simulink model "vegas01_4tap_1024ch_220_r8b"  (Simulink 7.5)
settings: Solver=ode45  StartTime=0.0  StopTime=128000  RelTol=1e-3
libraries: xbsIndex_r4 x12580, monroe_library x80, xbsReplacements_r4 x42, xps_library x34, casper_library_misc x27, dspsigops x16, simulink x7
(part 9 of 91)

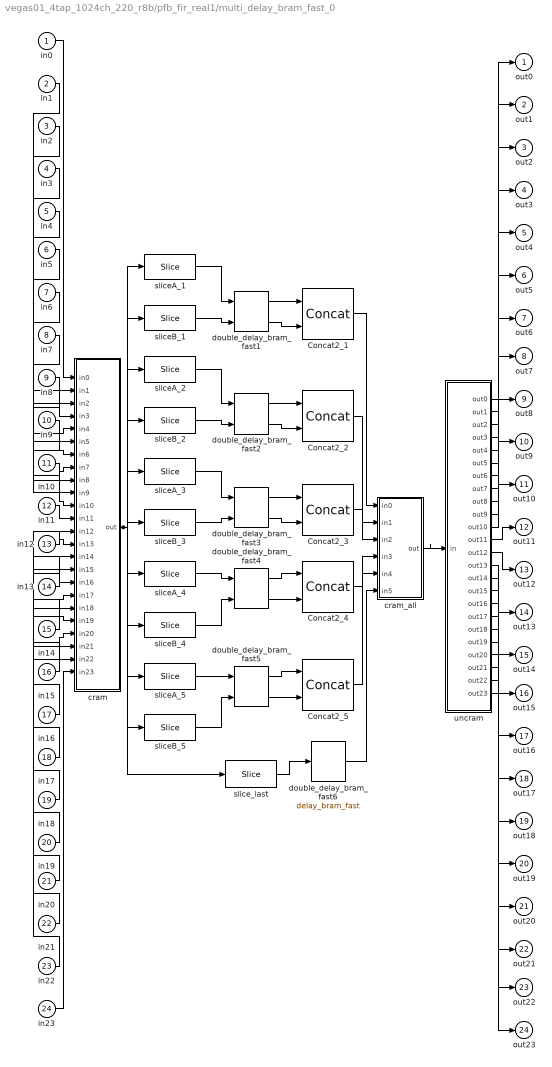
== system vegas01_4tap_1024ch_220_r8b/pfb_fir_real1/multi_delay_bram_fast_0 ==
  in0 : Inport
  in1 : Inport  Port=2
  in2 : Inport  Port=3
  in3 : Inport  Port=4
  in4 : Inport  Port=5
  in5 : Inport  Port=6
  in6 : Inport  Port=7
  in7 : Inport  Port=8
  in8 : Inport  Port=9
  in9 : Inport  Port=10
  in10 : Inport  Port=11
  in11 : Inport  Port=12
  in12 : Inport  Port=13
  in13 : Inport  Port=14
  in14 : Inport  Port=15
  in15 : Inport  Port=16
  in16 : Inport  Port=17
  in17 : Inport  Port=18
  in18 : Inport  Port=19
  in19 : Inport  Port=20
  in20 : Inport  Port=21
  in21 : Inport  Port=22
  in22 : Inport  Port=23
  in23 : Inport  Port=24
  Concat2_{1..5} (5 alike) : xbsIndex_r4/Concat  num_inputs=2  dbl_ovrd=off  block_type=concat
  cram : Subsystem
  cram_all : Subsystem
  double_delay_bram_fast{1..5} (5 alike) : monroe_library/double_delay_bram_fast  SystemSampleTime=-1  delay_len=256  bram_latency=2  register_counter=1
  double_delay_bram_fast6 : monroe_library/delay_bram_fast  SystemSampleTime=-1  delay_len=256  bram_latency=2  memory_type=Block RAM  register_counter=1
  sliceA_1 : xbsIndex_r4/Slice  nbits=18  boolean_output=off  mode=Upper Bit Location + Width  bit1=-0  base1=MSB of Input  bit0=-0  base0=MSB of Input  dbl_ovrd=off  block_type=slice
  sliceA_2 : xbsIndex_r4/Slice  nbits=18  boolean_output=off  mode=Upper Bit Location + Width  bit1=-36  base1=MSB of Input  bit0=-36  base0=MSB of Input  dbl_ovrd=off  block_type=slice
  sliceA_3 : xbsIndex_r4/Slice  nbits=18  boolean_output=off  mode=Upper Bit Location + Width  bit1=-72  base1=MSB of Input  bit0=-72  base0=MSB of Input  dbl_ovrd=off  block_type=slice
  sliceA_4 : xbsIndex_r4/Slice  nbits=18  boolean_output=off  mode=Upper Bit Location + Width  bit1=-108  base1=MSB of Input  bit0=-108  base0=MSB of Input  dbl_ovrd=off  block_type=slice
  sliceA_5 : xbsIndex_r4/Slice  nbits=18  boolean_output=off  mode=Upper Bit Location + Width  bit1=-144  base1=MSB of Input  bit0=-144  base0=MSB of Input  dbl_ovrd=off  block_type=slice
  sliceB_1 : xbsIndex_r4/Slice  nbits=18  boolean_output=off  mode=Upper Bit Location + Width  bit1=-18  base1=MSB of Input  bit0=-18  base0=MSB of Input  dbl_ovrd=off  block_type=slice
  sliceB_2 : xbsIndex_r4/Slice  nbits=18  boolean_output=off  mode=Upper Bit Location + Width  bit1=-54  base1=MSB of Input  bit0=-54  base0=MSB of Input  dbl_ovrd=off  block_type=slice
  sliceB_3 : xbsIndex_r4/Slice  nbits=18  boolean_output=off  mode=Upper Bit Location + Width  bit1=-90  base1=MSB of Input  bit0=-90  base0=MSB of Input  dbl_ovrd=off  block_type=slice
  sliceB_4 : xbsIndex_r4/Slice  nbits=18  boolean_output=off  mode=Upper Bit Location + Width  bit1=-126  base1=MSB of Input  bit0=-126  base0=MSB of Input  dbl_ovrd=off  block_type=slice
  sliceB_5 : xbsIndex_r4/Slice  nbits=18  boolean_output=off  mode=Upper Bit Location + Width  bit1=-162  base1=MSB of Input  bit0=-162  base0=MSB of Input  dbl_ovrd=off  block_type=slice
  slice_last : xbsIndex_r4/Slice  nbits=12  boolean_output=off  mode=Lower Bit Location + Width  bit1=0  base1=LSB of Input  bit0=0  base0=LSB of Input  dbl_ovrd=off  block_type=slice
  uncram : Subsystem
  out0 : Outport
  out1 : Outport  Port=2
  out2 : Outport  Port=3
  out3 : Outport  Port=4
  out4 : Outport  Port=5
  out5 : Outport  Port=6
  out6 : Outport  Port=7
  out7 : Outport  Port=8
  out8 : Outport  Port=9
  out9 : Outport  Port=10
  out10 : Outport  Port=11
  out11 : Outport  Port=12
  out12 : Outport  Port=13
  out13 : Outport  Port=14
  out14 : Outport  Port=15
  out15 : Outport  Port=16
  out16 : Outport  Port=17
  out17 : Outport  Port=18
  out18 : Outport  Port=19
  out19 : Outport  Port=20
  out20 : Outport  Port=21
  out21 : Outport  Port=22
  out22 : Outport  Port=23
  out23 : Outport  Port=24
  wires:
  cram/1(out) -> slice_last/1, sliceB_5/1, sliceA_5/1, sliceB_4/1, sliceA_4/1, sliceB_3/1, sliceA_3/1, sliceB_2/1, sliceA_2/1, sliceB_1/1, sliceA_1/1
  uncram/24(out23) -> out23/1
  uncram/23(out22) -> out22/1
  uncram/22(out21) -> out21/1
  uncram/21(out20) -> out20/1
  uncram/20(out19) -> out19/1
  uncram/19(out18) -> out18/1
  uncram/18(out17) -> out17/1
  uncram/17(out16) -> out16/1
  uncram/16(out15) -> out15/1
  uncram/15(out14) -> out14/1
  uncram/14(out13) -> out13/1
  uncram/13(out12) -> out12/1
  uncram/12(out11) -> out11/1
  uncram/11(out10) -> out10/1
  uncram/10(out9) -> out9/1
  uncram/9(out8) -> out8/1
  uncram/8(out7) -> out7/1
  uncram/7(out6) -> out6/1
  uncram/6(out5) -> out5/1
  uncram/5(out4) -> out4/1
  uncram/4(out3) -> out3/1
  uncram/3(out2) -> out2/1
  uncram/2(out1) -> out1/1
  uncram/1(out0) -> out0/1
  cram_all/1(out) -> uncram/1(in)
  double_delay_bram_fast6/1 -> cram_all/6(in5)
  Concat2_5/1 -> cram_all/5(in4)
  Concat2_4/1 -> cram_all/4(in3)
  Concat2_3/1 -> cram_all/3(in2)
  Concat2_2/1 -> cram_all/2(in1)
  Concat2_1/1 -> cram_all/1(in0)
  slice_last/1 -> double_delay_bram_fast6/1
  double_delay_bram_fast5/2 -> Concat2_5/2
  double_delay_bram_fast5/1 -> Concat2_5/1
  sliceB_5/1 -> double_delay_bram_fast5/2
  sliceA_5/1 -> double_delay_bram_fast5/1
  double_delay_bram_fast4/2 -> Concat2_4/2
  double_delay_bram_fast4/1 -> Concat2_4/1
  sliceB_4/1 -> double_delay_bram_fast4/2
  sliceA_4/1 -> double_delay_bram_fast4/1
  double_delay_bram_fast3/2 -> Concat2_3/2
  double_delay_bram_fast3/1 -> Concat2_3/1
  sliceB_3/1 -> double_delay_bram_fast3/2
  sliceA_3/1 -> double_delay_bram_fast3/1
  double_delay_bram_fast2/2 -> Concat2_2/2
  double_delay_bram_fast2/1 -> Concat2_2/1
  sliceB_2/1 -> double_delay_bram_fast2/2
  sliceA_2/1 -> double_delay_bram_fast2/1
  double_delay_bram_fast1/2 -> Concat2_1/2
  double_delay_bram_fast1/1 -> Concat2_1/1
  sliceB_1/1 -> double_delay_bram_fast1/2
  sliceA_1/1 -> double_delay_bram_fast1/1
  in23/1 -> cram/24(in23)
  in22/1 -> cram/23(in22)
  in21/1 -> cram/22(in21)
  in20/1 -> cram/21(in20)
  in19/1 -> cram/20(in19)
  in18/1 -> cram/19(in18)
  in17/1 -> cram/18(in17)
  in16/1 -> cram/17(in16)
  in15/1 -> cram/16(in15)
  in14/1 -> cram/15(in14)
  in13/1 -> cram/14(in13)
  in12/1 -> cram/13(in12)
  in11/1 -> cram/12(in11)
  in10/1 -> cram/11(in10)
  in9/1 -> cram/10(in9)
  in8/1 -> cram/9(in8)
  in7/1 -> cram/8(in7)
  in6/1 -> cram/7(in6)
  in5/1 -> cram/6(in5)
  in4/1 -> cram/5(in4)
  in3/1 -> cram/4(in3)
  in2/1 -> cram/3(in2)
  in1/1 -> cram/2(in1)
  in0/1 -> cram/1(in0)

== system vegas01_4tap_1024ch_220_r8b/pfb_fir_real3/coeff_gen_0_7 ==
  sync : Inport
  Counter0 : xbsIndex_r4/Counter  cnt_type=Free Running  cnt_to=Inf  operation=Up  start_count=8  cnt_by_val=1  arith_type=Unsigned  n_bits=8  bin_pt=0  load_pin=off  rst=on  en=off  explicit_period=on  period=1  dbl_ovrd=off  use_behavioral_HDL=off  implementation=Fabric  block_type=counter
  Counter1 : xbsIndex_r4/Counter  cnt_type=Free Running  cnt_to=Inf  operation=Down  start_count=247  cnt_by_val=1  arith_type=Unsigned  n_bits=8  bin_pt=0  load_pin=off  rst=on  en=off  explicit_period=on  period=1  dbl_ovrd=off  use_behavioral_HDL=off  implementation=Fabric  block_type=counter
  Delay1 : xbsIndex_r4/Delay  rst=off  infoeditControl=Selection of Reset will increase slice count due to use of real FFs and instead of SRLs  en=off  latency=2  dbl_ovrd=off  reg_retiming=off  block_type=delay
  Delay2 : xbsIndex_r4/Delay  rst=off  infoeditControl=Selection of Reset will increase slice count due to use of real FFs and instead of SRLs  en=off  latency=1  dbl_ovrd=off  reg_retiming=off  block_type=delay
  Delay3 : xbsIndex_r4/Delay  rst=off  infoeditControl=Selection of Reset will increase slice count due to use of real FFs and instead of SRLs  en=off  latency=1  dbl_ovrd=off  reg_retiming=off  block_type=delay
  Delay4 : xbsIndex_r4/Delay  rst=off  infoeditControl=Selection of Reset will increase slice count due to use of real FFs and instead of SRLs  en=off  latency=2  dbl_ovrd=off  reg_retiming=off  block_type=delay
  coeff_ram_1 : xbsIndex_r4/Dual Port RAM  depth=256  initVector=[-3.1185374660155e-18 -0.000156568793162539 -0.000313829844983494 -0.000471865598633262 -0.000630759038071172 -0.000790593639144139 -0.000951453320173921 -0.00111342239204917 -0.00127658550783793 -0.00144102761193718 -0.00160683388877549 -0.00177408971108584 -0.00194288058776502 -0.00211329211133704 -0.00228540990503756 -0.00245931956953669 -0.00263510662931799 -0.00281285647873119 -0.00299265432773675 -0.00317458514736013 -0.00335873361487434 -0.00354518405872877 -0.00373402040324324 -0.00392532611308567 -0.00411918413755233 -0.00431567685466965 -0.00451488601513651 -0.00471689268612641 -0.00492177719496865 -0.00512961907272809 -0.00534049699770283 -0.00555448873885967 -0.00577167109922673 -0.00599211985926327 -0.00621590972022655 -0.00644311424755544 -0.00667380581429108 -0.00690805554455435 -0.00714593325710056 -0.0073875074089712 -0.00763284503926322 -0.00788201171303579 -0.00813507146537516 -0.00839208674563745 -0.00865311836189 -0.00891822542557158 -0.0091874652963913 -0.00946089352748732 -0.00973856381086471 -0.0100205279231336 -0.0103068356715673 -0.0105975348405007 -0.010892671138089 -0.0111922881434479 -0.0114964272541938 -0.0118051276344046 -0.012118426163022 -0.0124363573827139 -0.0127589534492176 -0.0130862440811825 -0.0134182565105339 -0.0137550154333747 -0.0140965429614476 -0.0144428585741738 -0.0147939790712908 -0.0151499185261055 -0.015510688239384 -0.0158762966938947 -0.0162467495096255 -0.0166220493996923 -0.0170021961269577 -0.0173871864613782 -0.0177770141380983 -0.0181716698163081 -0.0185711410388843 -0.0189754121928306 -0.019384464470535 -0.0197982758318616 -0.0202168209670949 -0.0206400712607505 -0.0210679947562723 -0.02150055612163 -0.021937716615834 -0.0223794340563839 -0.022825662787666 -0.0232763536503145 -0.0237314539515533 -0.0241909074365316 -0.0246546542606692 -0.0251226309630254 -0.0255947704407058 -0.0260710019243209 -0.0265512509545099 -0.0270354393595443 -0.0275234852340214 -0.0280153029186648 -0.02851080298124 -0.0290098921986007 -0.0295124735398754 -0.030018446150807 -0.0305277053392568 -0.0310401425618828 -0.0315556454120042 -0.032074097608661 -0.0325953789868802 -0.0331193654891569 -0.0336459291581604 -0.0341749381306741 -0.0347062566327772 -0.0352397449762773 -0.0357752595564017 -0.0363126528507542 -0.0368517734195453 -0.0373924659071026 -0.0379345710446673 -0.0384779256544837 -0.0390223626551864 -0.0395677110684921 -0.0401137960271989 -0.0406604387844996 -0.0412074567246124 -0.0417546633747318 -0.042301868418306 -0.0428488777096403 -0.0433954932898318 -0.0439415134040368 -0.0444867325200723 -0.0450309413483536 -0.0455739268631693 -0.0461154723252939 -0.0466553573059384 -0.0471933577120388 -0.0477292458128818 -0.0482627902680681 -0.0487937561568089 -0.0493219050085576 -0.0498469948349719 -0.0503687801632044 -0.0508870120705198 -0.0514014382202336 -0.05191180289897 -0.0524178470552337 -0.0529193083392921 -0.0534159211443621 -0.0539074166490959 -0.0543935228613613 -0.0548739646633084 -0.0553484638577182 -0.0558167392156239 -0.0562785065251991 -0.0567334786419044 -0.0571813655398841 -0.0576218743646051 -0.0580547094867273 -0.0584795725571982 -0.0588961625635602 -0.0593041758874615 -0.0597033063633591 -0.0600932453384034 -0.0604736817334929 -0.0608443021054873 -0.0612047907105668 -0.0615548295687251 -0.0618940985293826 -0.0622222753381076 -0.0625390357044313 -0.0628440533707418 -0.0631370001822442 -0.0634175461579711 -0.0636853595628278 -0.0639401069806586 -0.0641814533883164 -0.0644090622307204 -0.0646225954968852 -0.0648217137969047 -0.0650060764398726 -0.0651753415127238 -0.0653291659599763 -0.0654672056643582 -0.0655891155282986 -0.0656945495562658 -0.0657831609379319 -0.0658546021321458 -0.0659085249516942 -0.0659445806488293 -0.0659624200015456 -0.0659616934005828 -0.0659420509371349 -0.0659031424912438 -0.0658446178208569 -0.0657661266515254 -0.0656673187667229 -0.0655478440987611 -0.0654073528202804 -0.0652454954362926 -0.0650619228767521 -0.0648562865896345 -0.064628238634496 ...(+1157 chars)]  distributed_mem=Block RAM  init_a=0  init_b=0  rst_a=off  rst_b=off  en_a=off  en_b=off  latency=2  write_mode_A=No Read On Write  write_mode_B=No Read On Write  dbl_ovrd=off  optimize=Area  use_rpm=on  block_type=dpram
  coeff_ram_2 : xbsIndex_r4/Dual Port RAM  depth=256  initVector=[2.10535666547559e-17 0.00212900955948074 0.00429668515967571 0.00650315122411092 0.00874852581948401 0.0110329205850847 0.0133564406634076 0.0157191846319806 0.0181212444364309 0.0205627053248104 0.023043645783203 0.0255641374726353 0.0281242451673111 0.0307240266941921 0.0333635328739444 0.0360428074632712 0.0387618870986534 0.0415208012415143 0.044319572124833 0.0471582147012203 0.0500367365924796 0.0529551380406698 0.0559134118606878 0.0589115433943901 0.0619495104662685 0.0650272833406989 0.0681448246807793 0.0713020895087723 0.0744990251681692 0.0777355712873909 0.0810116597451406 0.0843272146374221 0.0876821522462404 0.0910763810099955 0.0945098014955858 0.0979823063722314 0.101493780387033 0.105044100342275 0.108633135074489 0.112260745435285 0.115926784273964 0.119631096421919 0.123373518678842 0.127153879800738 0.130972000489756 0.134827693385857 0.138720763060312 0.142651006011044 0.146618210659827 0.150622157351339 0.154662618354086 0.158739357863189 0.162852132005056 0.167000688843928 0.171184768390318 0.175404102611337 0.179658415442912 0.183947422803904 0.188270832612124 0.192628344802252 0.197019651345652 0.201444436272104 0.205902375693431 0.210393137829031 0.214916383033319 0.21947176382507 0.224058924918664 0.22867750325723 0.233327128047692 0.238007420797708 0.242717995354501 0.24745845794558 0.25222840722134 0.257027434299547 0.261855122811695 0.266711048951228 0.271594781523624 0.27650588199834 0.281443904562596 0.286408396177002 0.291398896633021 0.296414938612245 0.301456047747492 0.306521742685707 0.31161153515265 0.316724930019371 0.321861425370457 0.327020512574038 0.33220167635354 0.337404394861171 0.342628139753137 0.347872376266556 0.353136563298071 0.358420153484148 0.363722593283031 0.369043323058348 0.374381777164357 0.379737384032801 0.385109566261371 0.390497740703747 0.395901318561207 0.401319705475786 0.406752301624961 0.412198501817848 0.41765769559289 0.423129267317018 0.428612596286257 0.434107056827774 0.439612018403325 0.445126845714092 0.450650898806897 0.456183533181742 0.461724099900691 0.467271945698036 0.472826413091746 0.478386840496164 0.483952562335939 0.489522909161153 0.495097207763634 0.500674781294419 0.506254949382349 0.511837028253763 0.517420330853274 0.523004166965589 0.52858784333836 0.534170663806028 0.53975192941464 0.545330938547599 0.550906987052345 0.556479368367905 0.562047373653314 0.567610291916857 0.573167410146117 0.578718013438789 0.584261385134243 0.589796806945793 0.595323559093652 0.600840920438539 0.606348168615905 0.611844580170759 0.617329430693046 0.622801994953559 0.628261547040357 0.633707360495634 0.639138708453047 0.644554863775432 0.649955099192899 0.655338687441275 0.660704901400846 0.666053014235387 0.671382299531429 0.676692031437751 0.68198148480504 0.687249935325708 0.692496659673825 0.697720935645132 0.702922042297109 0.708099260089058 0.713251871022173 0.718379158779564 0.723480408866197 0.728554908748728 0.733601947995182 0.738620818414463 0.743610814195645 0.748571232047023 0.753501371334883 0.758400534221968 0.763268025805597 0.768103154255408 0.772905230950694 0.777673570617301 0.782407491464052 0.787106315318659 0.791769367763108 0.796395978268463 0.800985480329079 0.805537211596169 0.810050514010716 0.814524733935677 0.818959222287464 0.823353334666666 0.827706431487968 0.832017878109261 0.836287044959883 0.840513307667989 0.844696047186994 0.848834649921077 0.852928507849702 0.856977018651141 0.860979585824954 0.864935618813407 0.868844533121792 0.87270575043763 0.876518698748711 0.880282812459962 0.8839975325091 0.887662306481051 0.891276588721101 0.894839840446757 0.898351529858287 0.901811132247913 0.905218130107634 0.908572013235644 0.911872278841335 0.915118431648839 0.918309983999099 0.921446455950444 0.92452737537763 0.92755227806934 0.93052070782411 0.933432216544651 0.936286364330567 0.939082719569415 0.941820859026115 0.944500367930665 0.947120840064154 0.949681877843045 0.952183092401712 0.954624103673203 ...(+639 chars)]  distributed_mem=Block RAM  init_a=0  init_b=0  rst_a=off  rst_b=off  en_a=off  en_b=off  latency=2  write_mode_A=No Read On Write  write_mode_B=No Read On Write  dbl_ovrd=off  optimize=Area  use_rpm=on  block_type=dpram
  coeff_ram_3 : xbsIndex_r4/Dual Port RAM  depth=256  initVector=[0.99999996616597 0.999965122732456 0.999862763148446 0.99969290322501 0.999455569174354 0.999150797604323 0.998778635510719 0.998339140267444 0.997832379614464 0.997258431643602 0.996617384782167 0.995909337774408 0.995134399660817 0.994292689755268 0.993384337620019 0.992409483038553 0.991368275986299 0.990260876599208 0.989087455140225 0.987848191963625 0.986543277477267 0.98517291210273 0.983737306233386 0.982236680190373 0.980671264176525 0.979041298228237 0.977347032165284 0.975588725538626 0.973766647576178 0.971881077126593 0.969932302601044 0.967920621913036 0.965846342416255 0.963709780840474 0.961511263225527 0.959251124853375 0.956929710178262 0.954547372755009 0.952104475165433 0.949601388942924 0.947038494495202 0.944416181025259 0.94173484645052 0.938994897320233 0.936196748731109 0.933340824241245 0.930427555782326 0.927457383570154 0.924430756013512 0.921348129621381 0.918209968908548 0.915016746299614 0.911768942031431 0.90846704405399 0.905111547929788 0.901702956731696 0.898241780939348 0.894728538334085 0.891163753892475 0.887547959678431 0.883881694733966 0.88016550496859 0.8763999430474 0.872585568277877 0.868722946495407 0.864812649947588 0.860855257177304 0.85685135290464 0.852801527907634 0.848706378901905 0.844566508419199 0.840382524684857 0.836155041494258 0.831884678088254 0.827572059027625 0.8232178140666 0.818822578025451 0.814386990662214 0.809911696543554 0.805397344914799 0.800844589569198 0.79625408871641 0.791626504850263 0.786962504615826 0.782262758675808 0.777527941576326 0.772758731612076 0.767955810690928 0.763119864197992 0.758251580859177 0.753351652604278 0.74842077442962 0.743459644260307 0.738468962812079 0.73344943345285 0.728401762063917 0.723326656900904 0.718224828454464 0.713096989310765 0.707943854011803 0.70276613891557 0.69756456205611 0.692339843003496 0.687092702723761 0.68182386343882 0.676534048486405 0.671223982180056 0.665894389669201 0.660545996799345 0.655179529972417 0.649795716007294 0.644395282000545 0.638978955187421 0.633547462803117 0.628101531944358 0.622641889431319 0.617169261669924 0.611684374514551 0.606187953131173 0.60068072186097 0.595163404084448 0.589636722086077 0.584101396919502 0.578558148273344 0.57300769433762 0.567450751670829 0.561888035067706 0.556320257427704 0.550748129624207 0.545172360374529 0.5395936561107 0.534012720851096 0.528430256072918 0.522846960585568 0.517263530404928 0.511680658628599 0.506099035312095 0.500519347346046 0.494942278334421 0.489368508473805 0.483798714433753 0.478233569238249 0.472673742148295 0.467119898545652 0.461572699817766 0.456032803243897 0.450500861882474 0.444977524459708 0.43946343525948 0.433959234014528 0.428465555798959 0.422983030922102 0.417512284823734 0.412053937970694 0.406608605754903 0.401176898392824 0.395759420826362 0.390356772625254 0.384969547890928 0.379598335161898 0.374243717320666 0.368906271502193 0.36358656900392 0.358285175197385 0.353002649441433 0.347739544997053 0.342496408943841 0.337273782098116 0.332072198932707 0.326892187498411 0.321734269347151 0.316598959456849 0.311486766158009 0.306398191062043 0.301333728991348 0.296293867911136 0.29127908886304 0.286289865900504 0.281326666025966 0.276389949129841 0.271480167931326 0.266597767921017 0.261743187305372 0.256916856952999 0.252119200342801 0.247350633513975 0.242611565017871 0.237902395871715 0.23322351951422 0.228575321763057 0.223958180774225 0.219372467003304 0.214818543168595 0.210296764216169 0.205807477286797 0.201351021684797 0.19692772884877 0.192537922324248 0.188181917738244 0.183860022775706 0.17957253715788 0.175319752622567 0.171101952906299 0.166919413728398 0.162772402776951 0.158661179696668 0.154585996078645 0.150547095452006 0.146544713277443 0.142579076942623 0.138650405759487 0.134758910963406 0.130904795714212 0.127088255099082 0.123309476137273 0.119568637786708 0.11586591095239 0.112201458496647 0.108575435251201 0.10498798803104 0.101439255650094 0.0979293689387025 0.0944584507628602 ...(+630 chars)]  distributed_mem=Block RAM  init_a=0  init_b=0  rst_a=off  rst_b=off  en_a=off  en_b=off  latency=2  write_mode_A=No Read On Write  write_mode_B=No Read On Write  dbl_ovrd=off  optimize=Area  use_rpm=on  block_type=dpram
  coeff_ram_4 : xbsIndex_r4/Dual Port RAM  depth=256  initVector=[2.10398116186568e-17 -0.00208910129848476 -0.00413981519217956 -0.00615228497148969 -0.00812665998606917 -0.0100630955685824 -0.0119617529574151 -0.0138227992183588 -0.0156464071652944 -0.0174327552798972 -0.0191820276303907 -0.0208944137893728 -0.02257010875074 -0.0242093128457345 -0.0258122316581412 -0.0273790759386581 -0.0289100615184676 -0.0304054092220342 -0.0318653447791543 -0.0332900987362853 -0.0346799063671784 -0.0360350075828442 -0.0373556468408754 -0.0386420730541536 -0.0398945394989689 -0.0411133037225755 -0.0422986274502136 -0.0434507764916224 -0.0445700206470716 -0.0456566336129395 -0.0467108928868625 -0.0477330796724853 -0.0487234787838377 -0.049682378549365 -0.05061007071564 -0.0515068503507829 -0.0523730157476159 -0.0532088683265811 -0.0540147125384468 -0.0547908557668308 -0.0555376082305665 -0.0562552828859398 -0.0569441953288227 -0.057604663696731 -0.0582370085708326 -0.0588415528779333 -0.0594186217924666 -0.0599685426385132 -0.0604916447918784 -0.0609882595822519 -0.0614587201954768 -0.0619033615759541 -0.0623225203292089 -0.0627165346246425 -0.0630857440984982 -0.0634304897570651 -0.0637511138801446 -0.0640479599248067 -0.0643213724294594 -0.0645716969182567 -0.06479927980587 -0.0650044683026467 -0.0651876103201817 -0.0653490543773238 -0.0654891495066428 -0.0656082451613795 -0.0657066911229038 -0.0657848374087011 -0.0658430341809138 -0.0658816316554575 -0.0659009800117358 -0.065901429302976 -0.0658833293672071 -0.0658470297389021 -0.0657928795613065 -0.0657212274994732 -0.0656324216540255 -0.0655268094756689 -0.0654047376804711 -0.0652665521659314 -0.0651125979278587 -0.064943218978077 -0.064758758262979 -0.0645595575829447 -0.0643459575126456 -0.0641182973222507 -0.0638769148995536 -0.0636221466730374 -0.0633543275358952 -0.0630737907710226 -0.0627808679769988 -0.0624758889950725 -0.062159181837169 -0.0618310726149323 -0.0614918854698198 -0.0611419425042617 -0.0607815637139019 -0.0604110669209325 -0.0600307677085362 -0.0596409793564514 -0.0592420127776692 -0.0588341764562795 -0.0584177763864743 -0.0579931160127225 -0.0575604961711263 -0.0571202150319706 -0.0566725680434763 -0.0562178478767671 -0.05575634437206 -0.0552883444860887 -0.0548141322407688 -0.0543339886731146 -0.0538481917864134 -0.0533570165026674 -0.0528607346163095 -0.0523596147491997 -0.0518539223069101 -0.0513439194363028 -0.0508298649844081 -0.0503120144586076 -0.0497906199881273 -0.0492659302868452 -0.0487381906174182 -0.0482076427567307 -0.0476745249626697 -0.0471390719422284 -0.0466015148209414 -0.0460620811136535 -0.0455209946966228 -0.0449784757809613 -0.0444347408874128 -0.0438900028224681 -0.0433444706558188 -0.0427983496991485 -0.0422518414862616 -0.0417051437545469 -0.0411584504277772 -0.0406119516002399 -0.0400658335221989 -0.0395202785866835 -0.0389754653176024 -0.038431568359178 -0.0378887584666988 -0.0373472024985835 -0.0368070634097553 -0.0362685002463175 -0.0357316681415289 -0.0351967183130705 -0.0346637980615986 -0.0341330507705774 -0.0336046159073849 -0.0330786290256842 -0.0325552217690525 -0.0320345218758609 -0.0315166531853959 -0.0310017356452133 -0.030489885319718 -0.0299812143999574 -0.0294758312146207 -0.0289738402422336 -0.0284753421245379 -0.0279804336810455 -0.0274892079247559 -0.027001754079026 -0.0265181575955805 -0.0260385001736508 -0.0255628597802303 -0.0250913106714347 -0.0246239234149519 -0.0241607649135716 -0.0237018984297787 -0.0232473836113981 -0.0227972765182771 -0.0223516296499902 -0.021910491974553 -0.0214739089581295 -0.0210419225957184 -0.0206145714428038 -0.0201918906479528 -0.0197739119863465 -0.0193606638942276 -0.0189521715042477 -0.0185484566816996 -0.018149538061616 -0.0177554310867198 -0.0173661480462071 -0.0169816981153476 -0.0166020873958826 -0.016227318957206 -0.0158573928783077 -0.0154923062904633 -0.015132053420651 -0.0147766256356781 -0.0144260114869981 -0.0140801967562004 -0.013739164501153 -0.0134028951027801 -0.0130713663124559 -0.0127445532999939 -0.0124224287022143 ...(+1169 chars)]  distributed_mem=Block RAM  init_a=0  init_b=0  rst_a=off  rst_b=off  en_a=off  en_b=off  latency=2  write_mode_A=No Read On Write  write_mode_B=No Read On Write  dbl_ovrd=off  optimize=Area  use_rpm=on  block_type=dpram
  const_din : xbsIndex_r4/Constant  const=0  gui_display_data_type=Fixed-point  arith_type=Signed (2's comp)  n_bits=18  bin_pt=17  preci_type=Single  exp_width=8  frac_width=24  explicit_period=on  period=1  equ=P=C  opselect=C  inp2=PCIN>>17  opr=+  inp1=P  carry=CIN  dbl_ovrd=off  block_type=constant
  const_we : xbsIndex_r4/Constant  const=0  gui_display_data_type=Fixed-point  arith_type=Boolean  n_bits=16  bin_pt=14  preci_type=Single  exp_width=8  frac_width=24  explicit_period=on  period=1  equ=P=C  opselect=C  inp2=PCIN>>17  opr=+  inp1=P  carry=CIN  dbl_ovrd=off  block_type=constant
  cram_lower : Subsystem
  cram_upper : Subsystem
  sync_out : Outport
  coeff : Outport  Port=2
  coeff_rev : Outport  Port=3
  wires:
  sync/1 -> Counter1/1, Counter0/1, sync_out/1
  Counter0/1 -> coeff_ram_4/1, coeff_ram_3/1, Delay2/1, Delay1/1
  const_din/1 -> coeff_ram_4/5, coeff_ram_4/2, coeff_ram_3/5, coeff_ram_3/2, coeff_ram_2/5, coeff_ram_2/2, coeff_ram_1/5, coeff_ram_1/2
  const_we/1 -> coeff_ram_4/6, coeff_ram_4/3, coeff_ram_3/6, coeff_ram_3/3, coeff_ram_2/6, coeff_ram_2/3, coeff_ram_1/6, coeff_ram_1/3
  Counter1/1 -> Delay4/1, Delay3/1, coeff_ram_2/4, coeff_ram_1/4
  cram_lower/1(out) -> coeff_rev/1
  cram_upper/1(out) -> coeff/1
  coeff_ram_1/2 -> cram_lower/4(in3)
  coeff_ram_2/2 -> cram_lower/3(in2)
  coeff_ram_3/2 -> cram_lower/2(in1)
  coeff_ram_4/2 -> cram_lower/1(in0)
  coeff_ram_4/1 -> cram_upper/4(in3)
  coeff_ram_3/1 -> cram_upper/3(in2)
  coeff_ram_2/1 -> cram_upper/2(in1)
  coeff_ram_1/1 -> cram_upper/1(in0)
  Delay4/1 -> coeff_ram_4/4
  Delay3/1 -> coeff_ram_3/4
  Delay2/1 -> coeff_ram_2/1
  Delay1/1 -> coeff_ram_1/1
